simulink model "ofdm_txrx_supermimo_coded"  (Simulink 7.7)
settings: Solver=VariableStepDiscrete  SolverMode=SingleTasking  StartTime=0.0  StopTime=2*simOnly_simLength  RelTol=1e-3
PostLoadFcn:
  | ofdm_rx_supermimo_init
InitFcn:
  | ofdm_rx_supermimo_init
StartFcn:
  | ofdm_rx_supermimo_init
values from neighbouring files (symbols this model uses):
  ActionID_AFTransmit = 31    [ofdm_tx_supermimo_init.m]
  ActionID_Disabled = 0    [ofdm_tx_supermimo_init.m]
  ActionID_SetFlagA = 62    [ofdm_tx_supermimo_init.m]
  ActionID_SetFlagB = 61    [ofdm_tx_supermimo_init.m]
  BER_RAM_init_size = 2048    [ofdm_tx_supermimo_init.m]
  channel_h = [1 0; 1 0] * 0.3    [ofdm_tx_supermimo_init.m]
  CPLength = 16    [ofdm_tx_supermimo_init.m]
  longCorr_coef_i = [-4*real(LTS) 0]    [ofdm_rx_supermimo_init.m]
  longCorr_coef_q = [4*imag(LTS) 0]    [ofdm_rx_supermimo_init.m]
  max_CP_length = 16    [ofdm_tx_supermimo_init.m]
  max_num_baseRateSymbols = 31    [ofdm_tx_supermimo_init.m]
  max_num_subcarriers = 64    [ofdm_tx_supermimo_init.m]
  max_num_trainingSymbols = 15    [ofdm_tx_supermimo_init.m]
  max_numBytes = 16384    [ofdm_tx_supermimo_init.m]
  max_OFDM_symbols = 1023    [ofdm_tx_supermimo_init.m]
  modConstellation_qam16 = .75*[1 3 -1 -3]./3    [ofdm_tx_supermimo_init.m]
  modConstellation_qam64 = 0.875*[3 1 5 7 -3 -1 -5 -7]./7    [ofdm_tx_supermimo_init.m]
  modConstellation_qpsk = [1 -1]./sqrt(2)    [ofdm_tx_supermimo_init.m]
  numBaseRateSymbols = numBaseRateSymbols *2    [ofdm_tx_supermimo_init.m]
  numSubcarriers = 64    [ofdm_tx_supermimo_init.m]
  numTrainingSymbols = 2    [ofdm_tx_supermimo_init.m]
  pktTiming_count_payloadStart = pktTiming_count_lsStart + pktTiming_ls_num*pktTiming_ls_length    [ofdm_rx_supermimo_init.m]
  preamble = preamble_scale*[shortsyms_10 longsyms_2]    [ofdm_tx_supermimo_init.m]
  preamble_ROM = preamble_I_ROM + preamble_Q_ROM*2^16    [ofdm_tx_supermimo_init.m]
  RAM_init_size = max_numBytes    (= 16384)    [ofdm_tx_supermimo_init.m]
  RxDataScrambling_Seq = [40 198 78 63 82 173 102 245 48 111 172 115 147 230 216 93 72 65 62 2 205 242 122 90 128 83 105 97 73 10 5 252]    [ofdm_tx_supermimo_init.m]
  shortSymbol_time = shortSymbol_time(1:16).'    [ofdm_tx_supermimo_init.m]
  simOnly_numSamples = length(preamble)+( (numSubcarriers+CPLength)*(numTrainingSymbols + numBaseRateSymbols + numFullRateSymbols/2) )    [ofdm_tx_supermimo_init.m]
  train = [train train]    [ofdm_tx_supermimo_init.m]
  TxDataScrambling_Seq = [40 198 78 63 82 173 102 245 48 111 172 115 147 230 216 93 72 65 62 2 205 242 122 90 128 83 105 97 73 10 5 252]    [ofdm_tx_supermimo_init.m]
undefined symbols (not defined in the file or in its folder): AntA, AntB, BaseRate, Down, gcbh, numBuffs, numBytes, Up, xlBlockLoadCallback, xlGetNormalizedPeriod, xlipgetpartsetting
libraries: xbsIndex_r4 x5430
(part 6 of 49)

== system ofdm_txrx_supermimo_coded/OFDM Rx MIMO/Packet_Timing_Control ==
  Pkt Det : Inport
  Long CorrA : Inport  Port=2
  Done : Inport  Port=3
  ASR : xbsIndex_r4/Addressable Shift Register  infer_latency=on  depth=2  initVector=[0]  en=off  dbl_ovrd=off  use_behavioral_HDL=off  Optimization=Resource  use_rpm=off  block_type=addrsr
      settings: infoedit="Delay of  configurable length.  Any element  in the delay     line   can    be   addressed   and   driven    onto   the   output     port.<P><P>Hardware  notes: Implemented  using SRL16s.     If Virtex-4, Virtex-II or Spartan-3 devices are used, multiple SRLC16s are cascaded together."  xl_area=[13 0 0 25 0 0 0]  block_version=VER_STRING_GOES_HERE
  Assert : xbsIndex_r4/Assert  assert_type=on  type_source=Explicitly  gui_display_data_type=Fixed-point  arith_type=Boolean  n_bits=16  bin_pt=14  preci_type=Single  exp_width=8  frac_width=24  assert_rate=on  rate_source=Explicitly  period=4  output_port=on  block_type=assert
      settings: infoedit=Asserts a user-defined sample rate and/or type on a signal.<P><P>Hardware notes: In hardware this block costs nothing.  block_version=VER_STRING_GOES_HERE
  Constant1 : xbsIndex_r4/Constant  const=0  gui_display_data_type=Fixed-point  arith_type=Boolean  n_bits=1  bin_pt=0  preci_type=Single  exp_width=8  frac_width=24  explicit_period=off  period=1  equ=P=C  opselect=C  inp2=PCIN>>17  opr=+  inp1=P  carry=CIN  dbl_ovrd=off  block_type=constant
      settings: block_version=VER_STRING_GOES_HERE
  Constant11 : xbsIndex_r4/Constant  const=pktTiming_count_payloadStart-2  gui_display_data_type=Fixed-point  arith_type=Unsigned  n_bits=ceil(log2(pktTiming_count_payloadStart))  bin_pt=0  preci_type=Single  exp_width=8  frac_width=24  explicit_period=off  period=1  equ=P=C  opselect=C  inp2=PCIN>>17  opr=+  inp1=P  carry=CIN  dbl_ovrd=off  block_type=constant
      settings: block_version=VER_STRING_GOES_HERE
  Constant6 : xbsIndex_r4/Constant  const=pktTiming_count_payloadStart  gui_display_data_type=Fixed-point  arith_type=Unsigned  n_bits=ceil(log2(pktTiming_count_payloadStart))+1  bin_pt=0  preci_type=Single  exp_width=8  frac_width=24  explicit_period=off  period=1  equ=P=C  opselect=C  inp2=PCIN>>17  opr=+  inp1=P  carry=CIN  dbl_ovrd=off  block_type=constant
      settings: block_version=VER_STRING_GOES_HERE
  Convert1 : xbsIndex_r4/Convert  gui_display_data_type=Fixed-point  arith_type=Unsigned  n_bits=1  bin_pt=0  float_type=Single  exp_bits=8  fraction_bits=24  quantization=Truncate  overflow=Wrap  en=off  latency=0  dbl_ovrd=off  pipeline=off  block_type=convert
      settings: xl_area=[0, 0, 0, 0, 0, 0, 0]  block_version=VER_STRING_GOES_HERE
  Convert2 : xbsIndex_r4/Convert  gui_display_data_type=Fixed-point  arith_type=Unsigned  n_bits=16  bin_pt=0  float_type=Single  exp_bits=8  fraction_bits=24  quantization=Truncate  overflow=Wrap  en=off  latency=0  dbl_ovrd=off  pipeline=off  block_type=convert
      settings: xl_area=[0, 0, 0, 0, 0, 0, 0]  block_version=VER_STRING_GOES_HERE
  Copy_Downsample : Subsystem
  Counter : xbsIndex_r4/Counter  cnt_type=Free Running  cnt_to=Inf  operation=Up  start_count=0  cnt_by_val=1  arith_type=Unsigned  n_bits=pktTiming_controlCounter_bits  bin_pt=0  load_pin=on  rst=on  en=on  explicit_period=off  period=1  dbl_ovrd=off  use_behavioral_HDL=off  implementation=Fabric  use_rpm=on  block_type=counter
      settings: xl_area=[6 11 0 12 0 0 0]  block_version=VER_STRING_GOES_HERE
  Disable for Interrupts : Subsystem
  Edge Det : Subsystem
  Edge Det1 : Subsystem
  Ext Reset : Subsystem
  Fine_Pkt_det : Subsystem
  Inverter1 : xbsIndex_r4/Inverter  en=off  latency=0  dbl_ovrd=off  block_type=inv
      settings: xl_area=[0 0 0 0 0 0 0]  block_version=VER_STRING_GOES_HERE
  Inverter3 : xbsIndex_r4/Inverter  en=off  latency=0  dbl_ovrd=off  block_type=inv
      settings: xl_area=[0 0 0 0 0 0 0]  block_version=VER_STRING_GOES_HERE
  Logical2 : xbsIndex_r4/Logical  logical_function=AND  inputs=2  en=off  latency=0  precision=Full  arith_type=Unsigned  n_bits=8  bin_pt=2  align_bp=on  dbl_ovrd=off  block_type=logical
      settings: xl_area=[1 0 0 1 0 0 0]  block_version=VER_STRING_GOES_HERE
  Logical3 : xbsIndex_r4/Logical  logical_function=AND  inputs=2  en=off  latency=0  precision=Full  arith_type=Unsigned  n_bits=8  bin_pt=2  align_bp=on  dbl_ovrd=off  block_type=logical
      settings: xl_area=[1 0 0 1 0 0 0]  block_version=VER_STRING_GOES_HERE
  Logical4 : xbsIndex_r4/Logical  logical_function=AND  inputs=2  en=off  latency=0  precision=Full  arith_type=Unsigned  n_bits=8  bin_pt=2  align_bp=on  dbl_ovrd=off  block_type=logical
      settings: xl_area=[1 0 0 1 0 0 0]  block_version=VER_STRING_GOES_HERE
  Logical5 : xbsIndex_r4/Logical  logical_function=OR  inputs=6  en=off  latency=0  precision=Full  arith_type=Unsigned  n_bits=8  bin_pt=2  align_bp=on  dbl_ovrd=off  block_type=logical
      settings: xl_area=[1 0 0 1 0 0 0]  block_version=VER_STRING_GOES_HERE
  Logical6 : xbsIndex_r4/Logical  logical_function=AND  inputs=3  en=off  latency=0  precision=Full  arith_type=Unsigned  n_bits=8  bin_pt=2  align_bp=on  dbl_ovrd=off  block_type=logical
      settings: xl_area=[1 0 0 1 0 0 0]  block_version=VER_STRING_GOES_HERE
  Relational5 : xbsIndex_r4/Relational  mode=a>b  en=off  latency=0  dbl_ovrd=off  block_type=relational
      settings: xl_area=[6 0 0 11 0 0 0]  block_version=VER_STRING_GOES_HERE
  Relational6 : xbsIndex_r4/Relational  mode=a>b  en=off  latency=0  dbl_ovrd=off  block_type=relational
      settings: xl_area=[6 0 0 11 0 0 0]  block_version=VER_STRING_GOES_HERE
  Rx_PktDetReset : xbsIndex_r4/Gateway Out  inherit_from_input=off  hdl_port=on  timing_constraint=None  locs_specified=off  LOCs={}  UseAsDAC=off  DACChannel='1'  block_type=gatewayout
      settings: infoedit="Gateway out block.  Converts Xilinx fixed point inputs into ouputs of type Simulink integer, double, or fixed point.<P><P>Hardware notes:  In hardware these blocks become top level output ports or are discarded, depending on how they are configured."  xl_area=[0, 0, 0, 0, 0, 0, 0]  block_version=VER_STRING_GOES_HERE
  Rx_debug_PktDone : xbsIndex_r4/Gateway Out  inherit_from_input=off  hdl_port=on  timing_constraint=None  locs_specified=off  LOCs={}  UseAsDAC=off  DACChannel='1'  block_type=gatewayout
      settings: infoedit="Gateway out block.  Converts Xilinx fixed point inputs into ouputs of type Simulink integer, double, or fixed point.<P><P>Hardware notes:  In hardware these blocks become top level output ports or are discarded, depending on how they are configured."  xl_area=[0, 0, 0, 0, 0, 0, 0]  block_version=VER_STRING_GOES_HERE
  Rx_debug_payload : xbsIndex_r4/Gateway Out  inherit_from_input=off  hdl_port=on  timing_constraint=None  locs_specified=off  LOCs={}  UseAsDAC=off  DACChannel='1'  block_type=gatewayout
      settings: infoedit="Gateway out block.  Converts Xilinx fixed point inputs into ouputs of type Simulink integer, double, or fixed point.<P><P>Hardware notes:  In hardware these blocks become top level output ports or are discarded, depending on how they are configured."  xl_area=[0 0 0 0 1 0 0]  block_version=VER_STRING_GOES_HERE
  S-R Latch : Subsystem
  S-R Latch1 : Subsystem
  Up Sample : xbsIndex_r4/Up Sample  sample_ratio=4  copy_samples=on  en=off  latency=0  dbl_ovrd=off  block_type=usamp
      settings: infoedit="Up samples input data.  Inserted values  can be zeros or copies of the most recent input sample.<P><P>Hardware notes: No hardware is needed if inserted values are copies of the input sample; otherwise, a mux and single bit flip-flop are used."  block_version=8.2.02
  Up Sample1 : xbsIndex_r4/Up Sample  sample_ratio=2  copy_samples=on  en=off  latency=0  dbl_ovrd=off  block_type=usamp
      settings: infoedit="Up samples input data.  Inserted values  can be zeros or copies of the most recent input sample.<P><P>Hardware notes: No hardware is needed if inserted values are copies of the input sample; otherwise, a mux and single bit flip-flop are used."  block_version=8.2.02
  Up Sample2 : xbsIndex_r4/Up Sample  sample_ratio=4  copy_samples=on  en=off  latency=0  dbl_ovrd=off  block_type=usamp
      settings: infoedit="Up samples input data.  Inserted values  can be zeros or copies of the most recent input sample.<P><P>Hardware notes: No hardware is needed if inserted values are copies of the input sample; otherwise, a mux and single bit flip-flop are used."  block_version=8.2.02
  Payload_Preamble : Outport
  PktReset : Outport  Port=2
  PktDetected : Outport  Port=3
  tag scope: RxPktDone=global, RxPktTiming_SampleCount=global
  wires:
  Convert1/1 -> Up Sample2/1
  Copy_Downsample/1(Q) -> Logical5/6, Disable for Interrupts/1(PktDone)
  Assert/1 -> S-R Latch1/2(R), Counter/3, S-R Latch/2(R), PktReset/1, Up Sample/1
  Disable for Interrupts/1(Det Disabled) -> Logical5/5
  Done/1 -> Copy_Downsample/1(D), Up Sample1/1
  S-R Latch1/1(Q) -> Counter/4, PktDetected/1, Logical2/1
  S-R Latch/1(Q) -> Inverter3/1, Edge Det1/1(D)
  Edge Det1/1(Q) -> Counter/1
  Convert2/1 -> Counter/2
  Logical2/1 -> S-R Latch/1(S)
  Logical6/1 -> Logical5/2
  [regRx_reqLongCorr] -> Logical6/3
  Inverter3/1 -> Logical6/2
  Relational6/1 -> Logical6/1
  Constant11/1 -> Relational6/2
  [rx_global_reset_DV2] -> Logical5/1
  Logical5/1 -> Assert/1
  Logical3/1 -> Convert1/1, Payload_Preamble/1
  Relational5/1 -> Logical3/1
  Inverter1/1 -> Logical3/2
  [rx_global_reset_DV2] -> Inverter1/1
  [regRx_PktDet_Delay] -> ASR/2
  ASR/1 -> Edge Det/1(D)
  Pkt Det/1 -> ASR/1
  Constant6/1 -> Relational5/2
  Edge Det/1(Q) -> S-R Latch1/1(S)
  Counter/1 -> Relational5/1, Relational6/1, Fine_Pkt_det/1(Samp Count), [RxPktTiming_SampleCount]
  Fine_Pkt_det/1(Det) -> Logical2/2
  Up Sample/1 -> Rx_PktDetReset/1
  Up Sample1/1 -> Rx_debug_PktDone/1, [RxPktDone]
  Up Sample2/1 -> Rx_debug_payload/1
  Logical4/1 -> Logical5/3
  [Tx_Running] -> Logical4/1
  [regRx_forceDisableOnTx] -> Logical4/2
  Ext Reset/1(Rst) -> Logical5/4
  [regRx_PktDet_LongCorr_CountLoad] -> Convert2/1
  Long CorrA/1 -> Fine_Pkt_det/2(Long CorrA)

== system ofdm_txrx_supermimo_coded/OFDM Rx MIMO/Rx Reset Logic ==
  Constant : Constant  Value=0
  Convert : xbsIndex_r4/Convert  gui_display_data_type=Fixed-point  arith_type=Boolean  n_bits=8  bin_pt=6  float_type=Single  exp_bits=8  fraction_bits=24  quantization=Truncate  overflow=Wrap  en=off  latency=0  dbl_ovrd=off  pipeline=off  block_type=convert
      settings: xl_area=[0, 0, 0, 0, 0, 0, 0]  block_version=VER_STRING_GOES_HERE
  Down Sample6 : xbsIndex_r4/Down Sample  sample_ratio=2  sample_phase="Last Value of Frame  (most efficient)"  en=off  latency=1  dbl_ovrd=off  block_type=dsamp
      settings: block_version=VER_STRING_GOES_HERE
  Logical1 : xbsIndex_r4/Logical  logical_function=OR  inputs=2  en=off  latency=0  precision=Full  arith_type=Unsigned  n_bits=8  bin_pt=2  align_bp=on  dbl_ovrd=off  block_type=logical
      settings: xl_area=[1 0 0 1 0 0 0]  block_version=VER_STRING_GOES_HERE
  Rx_Reset : xbsIndex_r4/Gateway In  gui_display_data_type=Fixed-point  arith_type=Boolean  n_bits=6  bin_pt=0  preci_type=Single  exp_width=8  frac_width=24  quantization="Round  (unbiased: +/- Inf)"  overflow=Saturate  period=4  dbl_ovrd=off  timing_constraint=None  locs_specified=off  LOCs={}  inherit_from_input=off  UseAsADC=off  ADCChannel='1'  hdl_port=on  block_type=gatewayin
      settings: infoedit="Gateway in block.  Converts inputs of type Simulink integer, double and fixed point to  Xilinx fixed point type.<P><P>Hardware notes:  In hardware these blocks become top level input ports."  xl_area=[0 0 0 0 1 0 0]  block_version=VER_STRING_GOES_HERE
  tag scope: rx_global_reset=global, rx_global_reset_DV2=global
  wires:
  [regRx_GlobalReset] -> Logical1/1
  Logical1/1 -> [rx_global_reset], Down Sample6/1
  Rx_Reset/1 -> Convert/1
  Convert/1 -> Logical1/2
  Down Sample6/1 -> [rx_global_reset_DV2]
  Constant/1 -> Rx_Reset/1

== system ofdm_txrx_supermimo_coded/OFDM Tx MIMO/IFFT ==
  Payload Done : Inport
  xn_I : Inport  Port=2
  xn_Q : Inport  Port=3
  Start : Inport  Port=4
  Pkt Done : Inport  Port=5
  Constant2 : xbsIndex_r4/Constant  const=0  gui_display_data_type=Fixed-point  arith_type=Unsigned  n_bits=1  bin_pt=0  preci_type=Single  exp_width=8  frac_width=24  explicit_period=off  period=1  equ=P=C  opselect=C  inp2=PCIN>>17  opr=+  inp1=P  carry=CIN  dbl_ovrd=off  block_type=constant
      settings: block_version=VER_STRING_GOES_HERE
  Constant3 : xbsIndex_r4/Constant  const=0  gui_display_data_type=Fixed-point  arith_type=Boolean  n_bits=1  bin_pt=0  preci_type=Single  exp_width=8  frac_width=24  explicit_period=off  period=1  equ=P=C  opselect=C  inp2=PCIN>>17  opr=+  inp1=P  carry=CIN  dbl_ovrd=off  block_type=constant
      settings: block_version=VER_STRING_GOES_HERE
  Constant4 : xbsIndex_r4/Constant  const=1  gui_display_data_type=Fixed-point  arith_type=Boolean  n_bits=1  bin_pt=0  preci_type=Single  exp_width=8  frac_width=24  explicit_period=off  period=1  equ=P=C  opselect=C  inp2=PCIN>>17  opr=+  inp1=P  carry=CIN  dbl_ovrd=off  block_type=constant
      settings: block_version=VER_STRING_GOES_HERE
  Constant6 : xbsIndex_r4/Constant  const=1  gui_display_data_type=Fixed-point  arith_type=Boolean  n_bits=1  bin_pt=0  preci_type=Single  exp_width=8  frac_width=24  explicit_period=off  period=1  equ=P=C  opselect=C  inp2=PCIN>>17  opr=+  inp1=P  carry=CIN  dbl_ovrd=off  block_type=constant
      settings: block_version=VER_STRING_GOES_HERE
  Counter : xbsIndex_r4/Counter  cnt_type=Free Running  cnt_to=Inf  operation=Up/Down  start_count=0  cnt_by_val=1  arith_type=Unsigned  n_bits=3  bin_pt=0  load_pin=off  rst=on  en=on  explicit_period=off  period=1  dbl_ovrd=off  use_behavioral_HDL=off  implementation=Fabric  use_rpm=off  block_type=counter
  "Fast Fourier Transform 7.1 " : xbsIndex_r4/Fast Fourier Transform 7.1   transform_length=64  implementation_options=pipelined_streaming_io  target_clock_frequency=40  target_data_throughput=50  run_time_configurable_transform_length=off  phase_factor_width=24  scaling_options=scaled  rounding_modes=convergent_rounding  output_ordering=bit_reversed_order  cyclic_prefix_insertion=off  ce=off  sclr=on  ovflo=on  input_data_offset=three_cycle_offset  memory_options_data=block_ram  memory_options_phase_factors=block_ram  number_of_stages_using_block_ram_for_data_and_phase_factors=1  memory_options_reorder=block_ram  memory_options_hybrid=off  complex_mult_type=use_mults_resources  butterfly_type=use_luts  channels=1  input_ordering=natural_order  input_width=16  simulation_type=external_xfix  simulation_model=fftv71_CModel:fftv71_cmodel  ip_name=Fast Fourier Transform  ip_version=7.1  dsptool_ready=true  wrapper_available=true  port_translation_map={ 'ce' => 'en', 'sclr' => 'rst' }  ipcore_fpga_part=xlipgetpartsetting(gcb, {'virtex4', 'xc4vsx35', '-10', 'ff668'})  block_type=xfft_v7_1
  IFFT Ovflo : Scope
      settings: TimeRange=392.7801724137931  YMin=-0.5  YMax=0.5  SaveName=ScopeData19
  Inverter3 : xbsIndex_r4/Inverter  en=off  latency=0  dbl_ovrd=off  block_type=inv
      settings: block_version=VER_STRING_GOES_HERE
  Logical1 : xbsIndex_r4/Logical  logical_function=AND  inputs=2  en=off  latency=0  precision=Full  arith_type=Unsigned  n_bits=16  bin_pt=0  align_bp=on  dbl_ovrd=off  block_type=logical
      settings: block_version=VER_STRING_GOES_HERE
  Logical2 : xbsIndex_r4/Logical  logical_function=XOR  inputs=2  en=off  latency=0  precision=Full  arith_type=Unsigned  n_bits=16  bin_pt=0  align_bp=on  dbl_ovrd=off  block_type=logical
      settings: block_version=VER_STRING_GOES_HERE
  Logical3 : xbsIndex_r4/Logical  logical_function=AND  inputs=2  en=off  latency=0  precision=Full  arith_type=Unsigned  n_bits=16  bin_pt=0  align_bp=on  dbl_ovrd=off  block_type=logical
      settings: block_version=VER_STRING_GOES_HERE
  Logical4 : xbsIndex_r4/Logical  logical_function=OR  inputs=2  en=off  latency=0  precision=Full  arith_type=Unsigned  n_bits=16  bin_pt=0  align_bp=on  dbl_ovrd=off  block_type=logical
      settings: block_version=VER_STRING_GOES_HERE
  Posedge2 : Subsystem
  Relational2 : xbsIndex_r4/Relational  mode=a=b  en=off  latency=0  dbl_ovrd=off  block_type=relational
      settings: xl_area=[2 0 0 4 0 0 0]  block_version=VER_STRING_GOES_HERE
  SR Latch : Subsystem
  done{1..8} (8 alike) : xbsIndex_r4/Gateway Out  inherit_from_input=off  hdl_port=off  timing_constraint=None  locs_specified=off  LOCs={}  UseAsDAC=off  DACChannel='1'  block_type=gatewayout
      settings: infoedit="Gateway out block.  Converts Xilinx fixed point inputs into ouputs of type Simulink integer, double, or fixed point.<P><P>Hardware notes:  In hardware these blocks become top level output ports or are discarded, depending on how they are configured."  xl_area=[0 0 0 0 1 0 0]  block_version=VER_STRING_GOES_HERE
  xn_Index : Outport
  Vout : Outport  Port=2
  xk_I : Outport  Port=3
  xk_Q : Outport  Port=4
  xk_Index : Outport  Port=5
  LastSym : Outport  Port=6
  wires:
  Constant3/1 -> "Fast Fourier Transform 7.1 "/4
  Constant4/1 -> "Fast Fourier Transform 7.1 "/5
  Constant6/1 -> "Fast Fourier Transform 7.1 "/7
  Logical4/1 -> Counter/2, SR Latch/2(R), "Fast Fourier Transform 7.1 "/8
  xn_I/1 -> done4/1, "Fast Fourier Transform 7.1 "/1
  "Fast Fourier Transform 7.1 "/1 -> xk_I/1, done1/1
  "Fast Fourier Transform 7.1 "/2 -> xk_Q/1, done2/1
  xn_Q/1 -> done5/1, "Fast Fourier Transform 7.1 "/2
  "Fast Fourier Transform 7.1 "/3 -> xn_Index/1, done3/1
  Logical1/1 -> Counter/1, Logical2/1
  "Fast Fourier Transform 7.1 "/4 -> xk_Index/1, done7/1
  "Fast Fourier Transform 7.1 "/7 -> Vout/1
  [Tx_FFTScaling] -> "Fast Fourier Transform 7.1 "/6
  "Fast Fourier Transform 7.1 "/8 -> Logical2/2
  Pkt Done/1 -> Logical4/2
  Inverter3/1 -> Logical1/2
  Start/1 -> Logical1/1, done6/1, "Fast Fourier Transform 7.1 "/3
  Logical2/1 -> Counter/3
  Constant2/1 -> Relational2/2
  Counter/1 -> Relational2/1
  Payload Done/1 -> Inverter3/1, Logical3/2
  Relational2/1 -> Logical3/1
  Logical3/1 -> Posedge2/1(D)
  SR Latch/1(Q) -> LastSym/1
  Posedge2/1(Q) -> SR Latch/1(S)
  [TxReset] -> Logical4/1
  done4/1 -> ?
  done5/1 -> ?
  done3/1 -> ?
  done6/1 -> ?
  done1/1 -> ?
  done2/1 -> ?
  done7/1 -> ?
  "Fast Fourier Transform 7.1 "/10 -> done8/1
  done8/1 -> IFFT Ovflo/1

== system ofdm_txrx_supermimo_coded/OFDM Tx MIMO/OutputBuffers ==
  IFFT Vout : Inport
  I : Inport  Port=2
  Q : Inport  Port=3
  Xk_index : Inport  Port=4
  Last Sym : Inport  Port=5
  1 Shift Reg : Subsystem
  1 Shift Reg2 : Subsystem
  Addr Gen : Subsystem
  Convert1 : xbsIndex_r4/Convert  gui_display_data_type=Fixed-point  arith_type="Signed  (2's comp)"  n_bits=14  bin_pt=13  float_type=Single  exp_bits=8  fraction_bits=24  quantization=Truncate  overflow=Flag as error  en=off  latency=0  dbl_ovrd=off  pipeline=off  block_type=convert
      settings: xl_area=[0 0 0 0 0 0 0]  block_version=VER_STRING_GOES_HERE
  Convert2 : xbsIndex_r4/Convert  gui_display_data_type=Fixed-point  arith_type="Signed  (2's comp)"  n_bits=14  bin_pt=13  float_type=Single  exp_bits=8  fraction_bits=24  quantization=Truncate  overflow=Flag as error  en=off  latency=0  dbl_ovrd=off  pipeline=off  block_type=convert
      settings: xl_area=[0 0 0 0 0 0 0]  block_version=VER_STRING_GOES_HERE
  Delay : xbsIndex_r4/Delay  rst=off  infoeditControl=Selection of Reset will increase slice count due to use of real FFs and instead of SRLs  en=off  latency=1  dbl_ovrd=off  reg_retiming=off  block_type=delay
      settings: infoedit=Hardware notes: A delay line is a chain, each link of which is an SRL16 followed by a flip-flop. If register retiming is enabled, the delay line is a chain of flip-flops.  block_version=VER_STRING_GOES_HERE
  Logical : xbsIndex_r4/Logical  logical_function=AND  inputs=2  en=off  latency=0  precision=Full  arith_type=Unsigned  n_bits=16  bin_pt=0  align_bp=on  dbl_ovrd=off  block_type=logical
      settings: block_version=VER_STRING_GOES_HERE
  Logical1 : xbsIndex_r4/Logical  logical_function=AND  inputs=2  en=off  latency=0  precision=Full  arith_type=Unsigned  n_bits=16  bin_pt=0  align_bp=on  dbl_ovrd=off  block_type=logical
      settings: block_version=VER_STRING_GOES_HERE
  Logical3 : xbsIndex_r4/Logical  logical_function=AND  inputs=2  en=off  latency=0  precision=Full  arith_type=Unsigned  n_bits=16  bin_pt=0  align_bp=on  dbl_ovrd=off  block_type=logical
      settings: block_version=VER_STRING_GOES_HERE
  Logical4 : xbsIndex_r4/Logical  logical_function=OR  inputs=2  en=off  latency=0  precision=Full  arith_type=Unsigned  n_bits=16  bin_pt=0  align_bp=on  dbl_ovrd=off  block_type=logical
      settings: block_version=VER_STRING_GOES_HERE
  Logical5 : xbsIndex_r4/Logical  logical_function=OR  inputs=2  en=off  latency=0  precision=Full  arith_type=Unsigned  n_bits=16  bin_pt=0  align_bp=on  dbl_ovrd=off  block_type=logical
      settings: block_version=VER_STRING_GOES_HERE
  Mem Control : Subsystem
  Mux1 : xbsIndex_r4/Mux  inputs=2  en=off  latency=0  precision=Full  arith_type=Unsigned  n_bits=16  bin_pt=14  quantization=Truncate  overflow=Wrap  dbl_ovrd=off  block_type=mux
  RAMs : Subsystem
  Rx Input : Scope  NumInputPorts=7
      settings: TimeRange=14600  YMin=-0.25~-0.25~-1~-1~0~0~0  YMax=0.25~0.25~1~1~1~3500~1  SaveName=ScopeData29
  Symbol Counter : xbsIndex_r4/Counter  cnt_type=Free Running  cnt_to=Inf  operation=Up  start_count=0  cnt_by_val=1  arith_type=Unsigned  n_bits=ceil(log2(max_OFDM_symbols+1))  bin_pt=0  load_pin=off  rst=on  en=on  explicit_period=off  period=1  dbl_ovrd=off  use_behavioral_HDL=off  implementation=Fabric  use_rpm=on  block_type=counter
      settings: xl_area=[4 7 0 7 0 0 0]  block_version=VER_STRING_GOES_HERE
  done{1..6} (6 alike) : xbsIndex_r4/Gateway Out  inherit_from_input=off  hdl_port=off  timing_constraint=None  locs_specified=off  LOCs={}  UseAsDAC=off  DACChannel='1'  block_type=gatewayout
      settings: infoedit="Gateway out block.  Converts Xilinx fixed point inputs into ouputs of type Simulink integer, double, or fixed point.<P><P>Hardware notes:  In hardware these blocks become top level output ports or are discarded, depending on how they are configured."  xl_area=[0 0 0 0 1 0 0]  block_version=VER_STRING_GOES_HERE
  negedge : Subsystem
  AntA_I : Outport
  AntA_Q : Outport  Port=2
  AntB_I : Outport  Port=3
  AntB_Q : Outport  Port=4
  Vout : Outport  Port=5
  Pkt Done : Outport  Port=6
  wires:
  Q/1 -> Convert1/1
  I/1 -> Convert2/1
  Convert2/1 -> RAMs/9(I)
  Convert1/1 -> RAMs/10(Q)
  Xk_index/1 -> Addr Gen/4(Xk Index)
  [LocalReset] -> Symbol Counter/1
  Logical/1 -> RAMs/1(A We)
  Logical1/1 -> RAMs/2(B We)
  RAMs/1(AntA_I) -> AntA_I/1
  RAMs/2(AntA_Q) -> AntA_Q/1
  RAMs/3(AntB_I) -> AntB_I/1
  RAMs/4(AntB_Q) -> AntB_Q/1
  Mem Control/1(weA) -> Logical/1
  Mem Control/2(weB) -> Logical1/2
  [LocalReset] -> Addr Gen/5(rst)
  Mem Control/4(BaseRateSym) -> Addr Gen/2(BaseRateSym)
  Symbol Counter/1 -> Mem Control/1(FFTsymNum)
  Addr Gen/2(OE#A) -> RAMs/3(A OE#), done3/1
  Addr Gen/3(OE#B) -> RAMs/4(B OE#), done6/1
  Addr Gen/4(Wr Addr) -> RAMs/5(Wr Addr), done4/1
  Addr Gen/5(Rd Addr) -> RAMs/6(Rd Addr), done5/1
  IFFT Vout/1 -> Logical/2, Logical1/1, negedge/1(D)
  negedge/1(Q) -> Symbol Counter/2, Addr Gen/3(SymWrDone)
  Mem Control/3(TrainingSym) -> Addr Gen/1(TrainingSym)
  [TxReset] -> Logical4/1
  Addr Gen/1(LastSamp) -> Logical3/2, 1 Shift Reg/3(en), 1 Shift Reg2/3(en)
  Logical3/1 -> Logical5/1, Delay/1
  RAMs/5(Rd Vout) -> Vout/1
  Addr Gen/6(Rd Vout) -> RAMs/7(Rd Vin), done1/1
  Logical4/1 -> [LocalReset]
  Delay/1 -> Logical5/2
  Logical5/1 -> Pkt Done/1, Logical4/2
  Addr Gen/7(Slot) -> RAMs/8(Slot), done2/1
  Last Sym/1 -> 1 Shift Reg/1(d)
  [TxReg_Alamouti_Mode] -> Mux1/1
  Mux1/1 -> Logical3/1
  1 Shift Reg/1(Q) -> Mux1/2, 1 Shift Reg2/1(d)
  [LocalReset] -> 1 Shift Reg/2(rst), 1 Shift Reg2/2(rst)
  1 Shift Reg2/1(Q) -> Mux1/3
  done4/1 -> Rx Input/1
  done5/1 -> Rx Input/2
  done1/1 -> Rx Input/3
  done2/1 -> Rx Input/4
  done3/1 -> Rx Input/5
  done6/1 -> Rx Input/6

== system ofdm_txrx_supermimo_coded/OFDM Tx MIMO/OutputMuxes ==
  note: Cheap output-enable circuit. Output registers are
      | brought out of reset on the first 0->1 transition in the
      | LSB of Pream Index. In other words, the outputs are
      | enabled when the preamble starts, and disabled when
      | the entire packet is finished.
  Pream Index : Inport
  AntA I : Inport  Port=2
  AntA Q : Inport  Port=3
  AntB I : Inport  Port=4
  AntB Q : Inport  Port=5
  vin : Inport  Port=6
  Pkt Done : Inport  Port=7
  Antenna Swapping : Subsystem
  Constant1 : xbsIndex_r4/Constant  const=0  gui_display_data_type=Fixed-point  arith_type=Boolean  n_bits=1  bin_pt=0  preci_type=Single  exp_width=8  frac_width=24  explicit_period=off  period=2  equ=P=C  opselect=C  inp2=PCIN>>17  opr=+  inp1=P  carry=CIN  dbl_ovrd=off  block_type=constant
      settings: block_version=VER_STRING_GOES_HERE
  Constant7 : xbsIndex_r4/Constant  const=1  gui_display_data_type=Fixed-point  arith_type=Boolean  n_bits=1  bin_pt=0  preci_type=Single  exp_width=8  frac_width=24  explicit_period=off  period=2  equ=P=C  opselect=C  inp2=PCIN>>17  opr=+  inp1=P  carry=CIN  dbl_ovrd=off  block_type=constant
      settings: block_version=VER_STRING_GOES_HERE
  Delay1 : xbsIndex_r4/Delay  rst=off  infoeditControl=Selection of Reset will increase slice count due to use of real FFs and instead of SRLs  en=off  latency=1  dbl_ovrd=off  reg_retiming=off  block_type=delay
      settings: infoedit=Hardware notes: A delay line is a chain, each link of which is an SRL16 followed by a flip-flop. If register retiming is enabled, the delay line is a chain of flip-flops.  block_version=VER_STRING_GOES_HERE
  Delay2 : xbsIndex_r4/Delay  rst=off  infoeditControl=Selection of Reset will increase slice count due to use of real FFs and instead of SRLs  en=off  latency=1  dbl_ovrd=off  reg_retiming=off  block_type=delay
      settings: infoedit=Hardware notes: A delay line is a chain, each link of which is an SRL16 followed by a flip-flop. If register retiming is enabled, the delay line is a chain of flip-flops.  block_version=VER_STRING_GOES_HERE
  Inverter : xbsIndex_r4/Inverter  en=off  latency=0  dbl_ovrd=off  block_type=inv
      settings: xl_area=[0, 0, 0, 0, 0, 0, 0]  block_version=VER_STRING_GOES_HERE
  LSB : xbsIndex_r4/Slice  nbits=1  boolean_output=on  mode=Lower Bit Location + Width  bit1=0  base1=MSB of Input  bit0=0  base0=LSB of Input  dbl_ovrd=off  block_type=slice
      settings: infoedit="Extracts a given range of bits from each input sample and presents it at the output.  The output type is ordinarily unsigned with binary point at zero, but can be Boolean when the slice is one bit wide.<P><P>Hardware notes: In hardware this block costs nothing."  block_version=VER_STRING_GOES_HERE
  Logical1 : xbsIndex_r4/Logical  logical_function=OR  inputs=2  en=off  latency=0  precision=Full  arith_type=Unsigned  n_bits=8  bin_pt=2  align_bp=on  dbl_ovrd=off  block_type=logical
      settings: xl_area=[1 0 0 1 0 0 0]  block_version=VER_STRING_GOES_HERE
  Logical2 : xbsIndex_r4/Logical  logical_function=OR  inputs=2  en=off  latency=0  precision=Full  arith_type=Unsigned  n_bits=8  bin_pt=2  align_bp=on  dbl_ovrd=off  block_type=logical
      settings: xl_area=[1 0 0 1 0 0 0]  block_version=VER_STRING_GOES_HERE
  Logical3 : xbsIndex_r4/Logical  logical_function=OR  inputs=2  en=off  latency=0  precision=Full  arith_type=Unsigned  n_bits=8  bin_pt=2  align_bp=on  dbl_ovrd=off  block_type=logical
      settings: xl_area=[1 0 0 1 0 0 0]  block_version=VER_STRING_GOES_HERE
  Mux{,1..3} (4 alike) : xbsIndex_r4/Mux  inputs=2  en=off  latency=0  precision=User Defined  arith_type="Signed  (2's comp)"  n_bits=14  bin_pt=13  quantization=Truncate  overflow=Wrap  dbl_ovrd=off  block_type=mux
      settings: xl_area=[7 0 0 14 0 0 0]  block_version=VER_STRING_GOES_HERE
  Payload Running : xbsIndex_r4/Register  init=0  rst=on  en=on  dbl_ovrd=off  block_type=register
      settings: xl_area=[1 1 0 0 0 0 0]  block_version=VER_STRING_GOES_HERE
  Payload Running1 : xbsIndex_r4/Register  init=1  rst=on  en=on  dbl_ovrd=off  block_type=register
      settings: xl_area=[1 1 0 0 0 0 0]  block_version=VER_STRING_GOES_HERE
  Posedge2 : Subsystem
  Preamble Generation : Subsystem
  Preambles : Scope  NumInputPorts=7
      settings: TimeRange=9328  YMin=-0.2~-0.25~-1~-1~0~0~0  YMax=0.15~0.15~1~1~1~3500~1  SaveName=ScopeData13
  Register{,1..3} (4 alike) : xbsIndex_r4/Register  init=0  rst=on  en=off  dbl_ovrd=off  block_type=register
      settings: xl_area=[0, 0, 0, 0, 0, 0, 0]  block_version=VER_STRING_GOES_HERE
  TxDone Logic : Subsystem
  TxRunning Outputs : Subsystem
  done1 : xbsIndex_r4/Gateway Out  inherit_from_input=off  hdl_port=off  timing_constraint=None  locs_specified=off  LOCs={}  UseAsDAC=off  DACChannel='1'  block_type=gatewayout
      settings: infoedit="Gateway out block.  Converts Xilinx fixed point inputs into ouputs of type Simulink integer, double, or fixed point.<P><P>Hardware notes:  In hardware these blocks become top level output ports or are discarded, depending on how they are configured."  xl_area=[0 0 0 0 1 0 0]  block_version=VER_STRING_GOES_HERE
  done4 : xbsIndex_r4/Gateway Out  inherit_from_input=off  hdl_port=off  timing_constraint=None  locs_specified=off  LOCs={}  UseAsDAC=off  DACChannel='1'  block_type=gatewayout
      settings: infoedit="Gateway out block.  Converts Xilinx fixed point inputs into ouputs of type Simulink integer, double, or fixed point.<P><P>Hardware notes:  In hardware these blocks become top level output ports or are discarded, depending on how they are configured."  xl_area=[0 0 0 0 1 0 0]  block_version=VER_STRING_GOES_HERE
  done5 : xbsIndex_r4/Gateway Out  inherit_from_input=off  hdl_port=off  timing_constraint=None  locs_specified=off  LOCs={}  UseAsDAC=off  DACChannel='1'  block_type=gatewayout
      settings: infoedit="Gateway out block.  Converts Xilinx fixed point inputs into ouputs of type Simulink integer, double, or fixed point.<P><P>Hardware notes:  In hardware these blocks become top level output ports or are discarded, depending on how they are configured."  xl_area=[0 0 0 0 1 0 0]  block_version=VER_STRING_GOES_HERE
  Payload : Outport
  " AntA I" : Outport  Port=2
  " AntA Q" : Outport  Port=3
  " AntB I" : Outport  Port=4
  " AntB Q" : Outport  Port=5
  tag scope: Tx_Running=global
  wires:
  Logical1/1 -> Mux/1, Mux1/1, Mux2/1, Mux3/1, Logical3/1
  Constant7/1 -> Payload Running/1
  TxDone Logic/1(Done) -> Payload Running/2, Delay1/1
  vin/1 -> Payload Running/3, Logical1/2
  AntA I/1 -> Mux/3
  AntA Q/1 -> Mux1/3
  Constant1/1 -> Payload Running1/1
  Payload Running1/1 -> Antenna Swapping/1(Rst), Register/2, Register1/2, Register2/2, Register3/2, Inverter/1
  LSB/1 -> Posedge2/1(D)
  Logical2/1 -> LSB/1, Antenna Swapping/2(PreamIndex), Preamble Generation/1(SampInd)
  Preamble Generation/2(AntA_Q) -> Mux1/2
  Register/1 -> " AntA I"/1
  Register1/1 -> " AntA Q"/1
  Mux2/1 -> Antenna Swapping/5(AntB_I)
  Mux3/1 -> Antenna Swapping/6(AntB_Q)
  Register2/1 -> " AntB I"/1
  Register3/1 -> " AntB Q"/1
  AntB I/1 -> Mux2/3
  AntB Q/1 -> Mux3/3
  Preamble Generation/3(AntB_I) -> Mux2/2
  Preamble Generation/4(AntB_Q) -> Mux3/2
  Payload Running/1 -> Logical1/1
  Delay1/1 -> Payload Running1/2
  Delay2/1 -> Payload/1
  Preamble Generation/1(AntA_I) -> Mux/2, done1/1
  Mux/1 -> Antenna Swapping/3(AntA_I)
  Mux1/1 -> Antenna Swapping/4(AntA_Q)
  Antenna Swapping/2(" AntA_I") -> Register/1
  Antenna Swapping/3(" AntA_Q") -> Register1/1
  Antenna Swapping/4(" AntB_I") -> Register2/1
  Antenna Swapping/5(" AntB_Q") -> Register3/1
  Pream Index/1 -> Logical2/2, done5/1
  [AF_PreambleIndex] -> Logical2/1, done4/1
  Posedge2/1(Q) -> Payload Running1/3
  Pkt Done/1 -> TxDone Logic/1(Pkt Done)
  Logical3/1 -> Delay2/1
  Antenna Swapping/1(AF Payload) -> Logical3/2
  Inverter/1 -> [Tx_Running], TxRunning Outputs/1(Tx)
  done4/1 -> Preambles/1
  done5/1 -> Preambles/2
  done1/1 -> Preambles/3

== system ofdm_txrx_supermimo_coded/OFDM Tx MIMO/Pre-Spin,  Filters & DACs ==
  Payload : Inport
  A_I : Inport  Port=2
  A_Q : Inport  Port=3
  B_I : Inport  Port=4
  B_Q : Inport  Port=5
  A*conj(B) : Subsystem
  "A*conj(B) " : Subsystem
  Interpolation : Subsystem
  Pre-CFO Sinusoid : Subsystem
  Scaling : Subsystem
  Tx : Scope  NumInputPorts=7
      settings: TimeRange=9328  YMin=-1~-1~-1~-1~-0.4~-0.4~0.25  YMax=1~1~1~1~0.4~0.5~1.5  SaveName=ScopeData9
  Tx_AntA_DAC_I : xbsIndex_r4/Gateway Out  inherit_from_input=off  hdl_port=on  timing_constraint=None  locs_specified=off  LOCs={}  UseAsDAC=off  DACChannel='1'  block_type=gatewayout
      settings: infoedit="Gateway out block.  Converts Xilinx fixed point inputs into ouputs of type Simulink integer, double, or fixed point.<P><P>Hardware notes:  In hardware these blocks become top level output ports or are discarded, depending on how they are configured."  block_version=10.1.3
  Tx_AntA_DAC_Q : xbsIndex_r4/Gateway Out  inherit_from_input=off  hdl_port=on  timing_constraint=None  locs_specified=off  LOCs={}  UseAsDAC=off  DACChannel='1'  block_type=gatewayout
      settings: infoedit="Gateway out block.  Converts Xilinx fixed point inputs into ouputs of type Simulink integer, double, or fixed point.<P><P>Hardware notes:  In hardware these blocks become top level output ports or are discarded, depending on how they are configured."  block_version=10.1.3
  Tx_AntB_DAC_I : xbsIndex_r4/Gateway Out  inherit_from_input=off  hdl_port=on  timing_constraint=None  locs_specified=off  LOCs={}  UseAsDAC=off  DACChannel='1'  block_type=gatewayout
      settings: infoedit="Gateway out block.  Converts Xilinx fixed point inputs into ouputs of type Simulink integer, double, or fixed point.<P><P>Hardware notes:  In hardware these blocks become top level output ports or are discarded, depending on how they are configured."  block_version=10.1.3
  Tx_AntB_DAC_Q : xbsIndex_r4/Gateway Out  inherit_from_input=off  hdl_port=on  timing_constraint=None  locs_specified=off  LOCs={}  UseAsDAC=off  DACChannel='1'  block_type=gatewayout
      settings: infoedit="Gateway out block.  Converts Xilinx fixed point inputs into ouputs of type Simulink integer, double, or fixed point.<P><P>Hardware notes:  In hardware these blocks become top level output ports or are discarded, depending on how they are configured."  block_version=10.1.3
  done{1..7} (7 alike) : xbsIndex_r4/Gateway Out  inherit_from_input=off  hdl_port=off  timing_constraint=None  locs_specified=off  LOCs={}  UseAsDAC=off  DACChannel='1'  block_type=gatewayout
      settings: infoedit="Gateway out block.  Converts Xilinx fixed point inputs into ouputs of type Simulink integer, double, or fixed point.<P><P>Hardware notes:  In hardware these blocks become top level output ports or are discarded, depending on how they are configured."  xl_area=[0 0 0 0 1 0 0]  block_version=VER_STRING_GOES_HERE
  " A_I" : Outport
  " A_Q" : Outport  Port=2
  " B_I" : Outport  Port=3
  " B_Q" : Outport  Port=4
  wires:
  Tx_AntA_DAC_I/1 -> " A_I"/1
  Tx_AntA_DAC_Q/1 -> " A_Q"/1
  Tx_AntB_DAC_I/1 -> " B_I"/1
  Tx_AntB_DAC_Q/1 -> " B_Q"/1
  Scaling/2(A_Q) -> Tx_AntA_DAC_Q/1, done7/1
  Scaling/4(B_Q) -> Tx_AntB_DAC_Q/1
  Scaling/3(B_I) -> Tx_AntB_DAC_I/1
  B_Q/1 -> "A*conj(B) "/4(Im[A])
  B_I/1 -> "A*conj(B) "/3(Re[A])
  Pre-CFO Sinusoid/1(I) -> A*conj(B)/4(Re[B]), "A*conj(B) "/1(Re[B])
  Pre-CFO Sinusoid/2(Q) -> A*conj(B)/3(Im[B]), "A*conj(B) "/2(Im[B])
  A_I/1 -> A*conj(B)/1(Re[A])
  A_Q/1 -> A*conj(B)/2(Im[A])
  Scaling/1(A_I) -> Tx_AntA_DAC_I/1, done1/1
  done4/1 -> Tx/1
  done5/1 -> Tx/2
  done3/1 -> Tx/3
  done6/1 -> Tx/4
  done2/1 -> Tx/5
  done1/1 -> Tx/6
  done7/1 -> Tx/7
  A*conj(B)/1(Re) -> done4/1, Interpolation/1("A_I ")
  A*conj(B)/2(Im) -> done5/1, Interpolation/2("A_Q ")
  "A*conj(B) "/1(Re) -> Interpolation/3("B_I ")
  "A*conj(B) "/2(Im) -> Interpolation/4("B_Q ")
  Interpolation/1(A_I) -> done3/1, Scaling/2("A_I ")
  Interpolation/3(B_I) -> Scaling/4("B_I ")
  Interpolation/4(B_Q) -> Scaling/5("B_Q ")
  Interpolation/2(A_Q) -> done6/1, Scaling/3("A_Q ")
  Payload/1 -> Scaling/1(Payload), done2/1

== system ofdm_txrx_supermimo_coded/OFDM Tx MIMO/Training_Data ==
  CPn_Data : Inport
  train_pay : Inport  Port=2
  BaseRate_En : Inport  Port=3
  FFT En : Inport  Port=4
  Start : Inport  Port=5
  packet_done : Inport  Port=6
  xn_index : Inport  Port=7
  Ant Selector : Subsystem
  Convert2 : xbsIndex_r4/Convert  gui_display_data_type=Fixed-point  arith_type="Signed  (2's comp)"  n_bits=16  bin_pt=15  float_type=Single  exp_bits=8  fraction_bits=24  quantization=Truncate  overflow=Wrap  en=off  latency=0  dbl_ovrd=off  pipeline=off  block_type=convert
      settings: xl_area=[0 0 0 0 0 0 0]  block_version=VER_STRING_GOES_HERE
  Convert3 : xbsIndex_r4/Convert  gui_display_data_type=Fixed-point  arith_type="Signed  (2's comp)"  n_bits=16  bin_pt=15  float_type=Single  exp_bits=8  fraction_bits=24  quantization=Truncate  overflow=Wrap  en=off  latency=0  dbl_ovrd=off  pipeline=off  block_type=convert
      settings: xl_area=[0 0 0 0 0 0 0]  block_version=VER_STRING_GOES_HERE
  Delay2 : xbsIndex_r4/Delay  rst=off  infoeditControl=Selection of Reset will increase slice count due to use of real FFs and instead of SRLs  en=off  latency=1  dbl_ovrd=off  reg_retiming=off  block_type=delay
      settings: infoedit=Hardware notes: A delay line is a chain, each link of which is an SRL16 followed by a flip-flop. If register retiming is enabled, the delay line is a chain of flip-flops.  xl_area=[1 1 0 0 0 0 0]  block_version=VER_STRING_GOES_HERE
  FlexibleMod : Subsystem
  Logical : xbsIndex_r4/Logical  logical_function=AND  inputs=3  en=off  latency=0  precision=Full  arith_type=Unsigned  n_bits=8  bin_pt=2  align_bp=on  dbl_ovrd=off  block_type=logical
      settings: xl_area=[0, 0, 0, 0, 0, 0, 0]  block_version=VER_STRING_GOES_HERE
  Logical1 : xbsIndex_r4/Logical  logical_function=OR  inputs=2  en=off  latency=0  precision=Full  arith_type=Unsigned  n_bits=8  bin_pt=2  align_bp=on  dbl_ovrd=off  block_type=logical
      settings: xl_area=[0, 0, 0, 0, 0, 0, 0]  block_version=VER_STRING_GOES_HERE
  Logical5 : xbsIndex_r4/Logical  logical_function=AND  inputs=2  en=off  latency=0  precision=Full  arith_type=Unsigned  n_bits=8  bin_pt=2  align_bp=on  dbl_ovrd=off  block_type=logical
      settings: xl_area=[0, 0, 0, 0, 0, 0, 0]  block_version=VER_STRING_GOES_HERE
  Training_Pilots : Subsystem
  tx_Q : Outport
  tx_I : Outport  Port=2
  Payload Done : Outport  Port=3
  wires:
  FlexibleMod/2(Q) -> Training_Pilots/3(Q_mod)
  FlexibleMod/1(I) -> Training_Pilots/2(I_mod)
  packet_done/1 -> FlexibleMod/2(packet_done), Logical1/1
  xn_index/1 -> FlexibleMod/1(ifft_index), Training_Pilots/1(Xn_index)
  train_pay/1 -> Delay2/1
  Logical/1 -> FlexibleMod/3(vin)
  Start/1 -> FlexibleMod/4(start)
  BaseRate_En/1 -> Ant Selector/1(BaseRate), Logical5/2
  CPn_Data/1 -> Ant Selector/2(CP#_Data), Logical/2
  Delay2/1 -> Logical5/1, Training_Pilots/4(train#), Logical/3
  Logical5/1 -> FlexibleMod/5(BaseRate_En)
  Ant Selector/1(Sel) -> FlexibleMod/6(ant_sel), Training_Pilots/5(antSel)
  FFT En/1 -> Logical/1
  Training_Pilots/1(tx_I) -> Convert3/1
  Training_Pilots/2(tx_Q) -> Convert2/1
  Convert3/1 -> tx_I/1
  Convert2/1 -> tx_Q/1
  Logical1/1 -> Training_Pilots/6(rst), Ant Selector/3(Rst)
  [TxReset] -> Logical1/2
  FlexibleMod/3(Payload Done) -> Payload Done/1

== system ofdm_txrx_supermimo_coded/OFDM Tx MIMO/Tx Reset Logic ==
  Logical : xbsIndex_r4/Logical  logical_function=OR  inputs=2  en=off  latency=0  precision=Full  arith_type=Unsigned  n_bits=16  bin_pt=0  align_bp=on  dbl_ovrd=off  block_type=logical
      settings: block_version=VER_STRING_GOES_HERE
  Pulse Generator1 : DiscretePulseGenerator  Period=1e6  PulseWidth=4  PhaseDelay=2750
  Step : Step  Before=1  After=0  SampleTime=0
  ToBool : xbsIndex_r4/Convert  gui_display_data_type=Fixed-point  arith_type=Boolean  n_bits=16  bin_pt=14  float_type=Single  exp_bits=8  fraction_bits=24  quantization=Truncate  overflow=Wrap  en=off  latency=0  dbl_ovrd=off  pipeline=off  block_type=convert
      settings: block_version=VER_STRING_GOES_HERE
  Tx_Reset : xbsIndex_r4/Gateway In  gui_display_data_type=Fixed-point  arith_type=Unsigned  n_bits=1  bin_pt=0  preci_type=Single  exp_width=8  frac_width=24  quantization="Round  (unbiased: +/- Inf)"  overflow=Saturate  period=4  dbl_ovrd=off  timing_constraint=None  locs_specified=off  LOCs={}  inherit_from_input=off  UseAsADC=off  ADCChannel='1'  hdl_port=on  block_type=gatewayin
      settings: infoedit="Gateway in block.  Converts inputs of type Simulink integer, double and fixed point to  Xilinx fixed point type.<P><P>Hardware notes:  In hardware these blocks become top level input ports."  xl_area=[0, 0, 0, 0, 0, 0, 0]  block_version=VER_STRING_GOES_HERE
  tag scope: TxReset=global
  wires:
  Tx_Reset/1 -> Logical/1
  Logical/1 -> ToBool/1
  ToBool/1 -> [TxReset]
  Step/1 -> Tx_Reset/1
  [TxReg_TxReset] -> Logical/2
